simulink model "TosAeb"  (R2021a)
settings: Solver=FixedStepDiscrete  StartTime=0.0  StopTime=inf  FixedStep=0.01  RelTol=1e-3
undefined symbols (not defined in the file or in its folder): ACCModSts, AccSts1, AEB_ObjectMovingOrientation, AEB_ObjectMovingState, CN_BTCActive, CN_CurrentGearR, CN_CurrentGearR_DHT, CN_PTCActive, CN_VDCActive, CN_VSC_EspOff, CN_VSC_FCW_AEB_Error, CN_VSC_FCW_AEB_NoDispay, CN_VSC_FCW_AEB_PerfmDgdtn, CN_VSC_FCW_AEB_TempUnAvlbl, CN_VSC_VehStandstill, CNaccelAvoidMax, CNaccelAvoidMin, CNbrakePedalPress, CNcomfortStrLeftAccelMax, CNcomfortStrLeftAccelMin, CNcomfortStrRightAccelMax, CNcomfortStrRightAccelMin, CNDrvDoorOpen, CNDrvModeEco, CNDrvModeNorm, CNDrvModeOther, CNDrvModeSport, CNDrvSBRreminder, CNNull, CNobjInvalidID, CNPassDoorOpen, CNPassSBRreminder, CNPEBSAccelNegInf, CNPEBSAccelNull, CNPEBSTimeInf, CNPEBSTimeNull, CNSTOPMAX, CNSTOPNULL, CNsubjWidth, CNSwtOFF, CNSwtON, CNTTCMAX, CNTTCNULL, CNVehConfigAEBCycNone, CNVehConfigAEBNone, CNVehConfigAEBPedNone, CNVehConfigFCWNone, CNVehConfigJANone, CollisionSeverity, FALSE, FCWSnvty, JACycObjCalcInfoDefaultParams, JAPedObjCalcInfoDefaultParams, JAVehObjCalcInfoDefaultParams, latCycObjCalcInfoDefaultParams, latPedObjCalcInfoDefaultParams, longObjCalcInfoDefaultParams, ModSts, movingStatus, ObjectType ... (+1112)
libraries: simulink x3031, AEBSFunctionLib x50, Ta_Library x33, AEBSStateMachineLib x23, Mylibrary x12, simulink_extras x7, ObjSelect_lib x3, dspstat3 x2
(part 81 of 102)

== system TosAeb/AEB_Objsel/TA_Processor/AEB_ObjSelect/TargetInfoCheck/Data_precess/TA_EffectCalculate/Obj_FilterFlgCalculate/Lat_ObjFilter/Lat_Objelse/Lat_ObjPre/Subsystem/LatDis_Cal/Width_Calculate ==
  Width : Inport
  Add : Sum
  Constant : Constant  Value=0.5
  Constant1 : Constant  Value=P_TA_PrmForVehWidth
  Product : Product
  Product1 : Product
  Width_Large : Outport
  Width_half : Outport  Port=2
  wires:
  Constant/1 -> Product/2, Product1/1
  Product1/1 -> Width_half/1, Add/2
  Product/1 -> Add/1
  Constant1/1 -> Product1/2
  Width/1 -> Product/1
  Add/1 -> Width_Large/1

== system TosAeb/AEB_Objsel/TA_Processor/AEB_ObjSelect/TargetInfoCheck/Data_precess/TA_EffectCalculate/Obj_FilterFlgCalculate/Lat_ObjFilter/Lat_Objelse/Lat_ObjPre/Subsystem/LatDis_Cal/others ==
  Action Port : ActionPort  ActionPortLabel=else
  Constant : Constant  Value=0  OutDataTypeStr=boolean
  Constant1 : Constant  Value=100  OutDataTypeStr=single
  Valid_Flg : Outport
  TTI : Outport  Port=2
  wires:
  Constant1/1 -> TTI/1
  Constant/1 -> Valid_Flg/1

== system TosAeb/AEB_Objsel/TA_Processor/AEB_ObjSelect/TargetInfoCheck/Data_precess/TA_EffectCalculate/Obj_FilterFlgCalculate/Lat_ObjFilter/Lat_Objelse/Lat_ObjPre/Subsystem/LatDis_Cal1/L2R ==
  dy : Inport
  Width_Large : Inport  Port=2
  Width_half : Inport  Port=3
  dx : Inport  Port=4
  ax : Inport  Port=5
  ay : Inport  Port=6
  vx : Inport  Port=7
  vy : Inport  Port=8
  Action Port : ActionPort
  Constant : Constant  Value=P_TA_PrmForVehLongth
  L2R_Calculate : MATLAB Function  TreatAsAtomicUnit=on
  Valid_Flg : Outport
  TTI : Outport  Port=2
  wires:
  Constant/1 -> L2R_Calculate/9(P_TA_PrmForVehLongth)
  L2R_Calculate/2(TTI) -> TTI/1
  L2R_Calculate/1(Valid_Flg) -> Valid_Flg/1
  Width_half/1 -> L2R_Calculate/3(Width_half)
  dx/1 -> L2R_Calculate/4(dx)
  Width_Large/1 -> L2R_Calculate/2(Width_Large)
  ax/1 -> L2R_Calculate/5(ax)
  dy/1 -> L2R_Calculate/1(dy)
  vy/1 -> L2R_Calculate/8(vy)
  ay/1 -> L2R_Calculate/6(ay)
  vx/1 -> L2R_Calculate/7(vx)

== system TosAeb/AEB_Objsel/TA_Processor/AEB_ObjSelect/TargetInfoCheck/Data_precess/TA_EffectCalculate/Obj_FilterFlgCalculate/Lat_ObjFilter/Lat_Objelse/Lat_ObjPre/Subsystem/LatDis_Cal1/Obj_PosnJudge ==
  Oreintation : Inport
  vy : Inport  Port=2
  Compare To Constant2 : simulink/Logic and Bit Operations/Compare To Constant  relop===  const=3  OutDataTypeStr=boolean
  Compare To Constant3 : simulink/Logic and Bit Operations/Compare To Constant  relop=>  const=P_TA_PrmForLatObjLatSpdThred  OutDataTypeStr=boolean
  Compare To Constant4 : simulink/Logic and Bit Operations/Compare To Constant  relop===  const=2  OutDataTypeStr=boolean
  Compare To Constant5 : simulink/Logic and Bit Operations/Compare To Constant  relop=>  const=P_TA_PrmForLatObjLatSpdThred  OutDataTypeStr=boolean
  Data Type Conversion : DataTypeConversion
  Data Type Conversion1 : DataTypeConversion
  Gain1 : Gain  Gain=-1  OutDataTypeStr=single
  Ground_1 : Ground
  Logical Operator : Logic  AllPortsSameDT=off  OutDataTypeStr=boolean
  Logical Operator1 : Logic  AllPortsSameDT=off  OutDataTypeStr=boolean
  Terminator_1 : Terminator
  L2R : Outport
  R2L : Outport  Port=2
  wires:
  Gain1/1 -> Terminator_1/1
  Ground_1/1 -> Gain1/1
  Data Type Conversion1/1 -> R2L/1
  Data Type Conversion/1 -> L2R/1
  Logical Operator/1 -> Data Type Conversion/1
  Logical Operator1/1 -> Data Type Conversion1/1
  Compare To Constant3/1 -> Logical Operator/2
  vy/1 -> Compare To Constant3/1, Compare To Constant5/1
  Compare To Constant4/1 -> Logical Operator1/1
  Compare To Constant5/1 -> Logical Operator1/2
  Compare To Constant2/1 -> Logical Operator/1
  Oreintation/1 -> Compare To Constant2/1, Compare To Constant4/1

== system TosAeb/AEB_Objsel/TA_Processor/AEB_ObjSelect/TargetInfoCheck/Data_precess/TA_EffectCalculate/Obj_FilterFlgCalculate/Lat_ObjFilter/Lat_Objelse/Lat_ObjPre/Subsystem/LatDis_Cal1/R2L ==
  dy : Inport
  Width_Large : Inport  Port=2
  Width_half : Inport  Port=3
  dx : Inport  Port=4
  ax : Inport  Port=5
  ay : Inport  Port=6
  vx : Inport  Port=7
  vy : Inport  Port=8
  Action Port : ActionPort
  Constant : Constant  Value=P_TA_PrmForVehLongth
  R2L_Calculate : MATLAB Function  TreatAsAtomicUnit=on
  Valid_Flg : Outport
  TTI : Outport  Port=2
  wires:
  Constant/1 -> R2L_Calculate/9(P_TA_PrmForVehLongth)
  R2L_Calculate/2(TTI) -> TTI/1
  R2L_Calculate/1(Valid_Flg) -> Valid_Flg/1
  vx/1 -> R2L_Calculate/7(vx)
  ay/1 -> R2L_Calculate/6(ay)
  vy/1 -> R2L_Calculate/8(vy)
  dy/1 -> R2L_Calculate/1(dy)
  ax/1 -> R2L_Calculate/5(ax)
  Width_Large/1 -> R2L_Calculate/2(Width_Large)
  dx/1 -> R2L_Calculate/4(dx)
  Width_half/1 -> R2L_Calculate/3(Width_half)

== system TosAeb/AEB_Objsel/TA_Processor/AEB_ObjSelect/TargetInfoCheck/Data_precess/TA_EffectCalculate/Obj_FilterFlgCalculate/Lat_ObjFilter/Lat_Objelse/Lat_ObjPre/Subsystem/LatDis_Cal1/Width_Calculate ==
  Width : Inport
  Add : Sum
  Constant : Constant  Value=0.5
  Constant1 : Constant  Value=P_TA_PrmForVehWidth
  Product : Product
  Product1 : Product
  Width_Large : Outport
  Width_half : Outport  Port=2
  wires:
  Add/1 -> Width_Large/1
  Width/1 -> Product/1
  Constant1/1 -> Product1/2
  Product/1 -> Add/1
  Product1/1 -> Add/2, Width_half/1
  Constant/1 -> Product1/1, Product/2

== system TosAeb/AEB_Objsel/TA_Processor/AEB_ObjSelect/TargetInfoCheck/Data_precess/TA_EffectCalculate/Obj_FilterFlgCalculate/Lat_ObjFilter/Lat_Objelse/Lat_ObjPre/Subsystem/LatDis_Cal1/others ==
  Action Port : ActionPort
  Constant : Constant  Value=0  OutDataTypeStr=boolean
  Constant1 : Constant  Value=100  OutDataTypeStr=single
  Valid_Flg : Outport
  TTI : Outport  Port=2
  wires:
  Constant/1 -> Valid_Flg/1
  Constant1/1 -> TTI/1

== system TosAeb/AEB_Objsel/TA_Processor/AEB_ObjSelect/TargetInfoCheck/Data_precess/TA_EffectCalculate/Obj_FilterFlgCalculate/Long_ObjFilter/OverlapCalculate/BoudingBoxCalculate_Else/Overlap_FlgCalculate/AbsPosn_InsectionFlg/ObjBoundingBox ==
  ObjLength : Inport  OutDataTypeStr=single
  ObjWidth : Inport  Port=2  OutDataTypeStr=single
  dxPreRefPointObj : Inport  Port=3  OutDataTypeStr=single
  dyPreRefPointObj : Inport  Port=4  OutDataTypeStr=single
  sincosCoef : Inport  Port=5
  Bus Creator : BusCreator  Inputs=5
  Mux : Mux
  Mux1 : Mux
  ObjLLCoordinate : Subsystem
  ObjLUCoordinate : Subsystem
  ObjRLCoordinate : Subsystem
  ObjRUCoordinate : Subsystem
  objDxPre : Outport
  objDyPre : Outport  Port=2
  wires:
  ObjLength/1 -> Bus Creator/1  "ObjLength"
  ObjWidth/1 -> Bus Creator/2  "ObjWidth"
  dxPreRefPointObj/1 -> Bus Creator/3  "dxPreRefPointObj"
  dyPreRefPointObj/1 -> Bus Creator/4  "dyPreRefPointObj"
  sincosCoef/1 -> Bus Creator/5  "sincosCoef"
  Bus Creator/1 -> ObjRLCoordinate/1(inputSignals), ObjRUCoordinate/1(inputSignals), ObjLLCoordinate/1(inputSignals), ObjLUCoordinate/1(inputSignals)
  ObjLUCoordinate/1(dxPreLUObj) -> [dxPreLUObj]  "dxPreLUObj"
  ObjLUCoordinate/2(dyPreLUobj) -> [dyPreLUObj]  "dyPreLUObj"
  ObjLLCoordinate/1(dxPre_LLObj) -> [dxPreLLObj]  "dxPreLLObj"
  ObjLLCoordinate/2(dyPreLLObj) -> [dyPreLLObj]  "dyPreLLObj"
  ObjRUCoordinate/1(dxPreRUObj) -> [dxPreRUObj]  "dxPreRUObj"
  ObjRUCoordinate/2(dyPreRUObj) -> [dyPreRUObj]  "dyPreRUObj"
  ObjRLCoordinate/1(dxPreRLObj) -> [dxPreRLObj]  "dxPreRLObj"
  ObjRLCoordinate/2(dyPreRLObj) -> [dyPreRLObj]  "dyPreRLObj"
  Mux/1 -> objDxPre/1
  Mux1/1 -> objDyPre/1
  [dxPreRUObj] -> Mux/1  "dxPreRUObj"
  [dxPreLUObj] -> Mux/2
  [dxPreLLObj] -> Mux/3
  [dxPreRLObj] -> Mux/4
  [dyPreRUObj] -> Mux1/1
  [dyPreLUObj] -> Mux1/2
  [dyPreLLObj] -> Mux1/3
  [dyPreRLObj] -> Mux1/4

== system TosAeb/AEB_Objsel/TA_Processor/AEB_ObjSelect/TargetInfoCheck/Data_precess/TA_EffectCalculate/Obj_FilterFlgCalculate/Long_ObjFilter/OverlapCalculate/BoudingBoxCalculate_Else/Overlap_FlgCalculate/AbsPosn_InsectionFlg/Overlap_Judgment ==
  subjDxPre : Inport
  subjDyPre : Inport  Port=2
  objDxPre : Inport  Port=3
  objDyPre : Inport  Port=4
  MinMax{,2,4,6} (4 alike) : MinMax
  MinMax{1,3,5,7} (4 alike) : MinMax  Function=max
  Overlap_FlgCalculate : MATLAB Function  TreatAsAtomicUnit=on
  Abs_OverlapFlg : Outport
  wires:
  subjDxPre/1 -> MinMax1/1, MinMax/1
  subjDyPre/1 -> MinMax2/1, MinMax3/1
  objDxPre/1 -> MinMax4/1, MinMax5/1
  objDyPre/1 -> MinMax6/1, MinMax7/1
  MinMax3/1 -> Overlap_FlgCalculate/3(Obj_y_Max)
  MinMax2/1 -> Overlap_FlgCalculate/4(Obj_y_Min)
  MinMax1/1 -> Overlap_FlgCalculate/1(Obj_x_Max)
  MinMax/1 -> Overlap_FlgCalculate/2(Obj_x_Min)
  MinMax5/1 -> Overlap_FlgCalculate/5(Subj_x_Max)
  MinMax4/1 -> Overlap_FlgCalculate/6(Subj_x_Min)
  MinMax7/1 -> Overlap_FlgCalculate/7(Subj_y_Max)
  MinMax6/1 -> Overlap_FlgCalculate/8(Subj_y_Min)
  Overlap_FlgCalculate/1(Overlap_Flg) -> Abs_OverlapFlg/1

== system TosAeb/AEB_Objsel/TA_Processor/AEB_ObjSelect/TargetInfoCheck/Data_precess/TA_EffectCalculate/Obj_FilterFlgCalculate/Long_ObjFilter/OverlapCalculate/BoudingBoxCalculate_Else/Overlap_FlgCalculate/AbsPosn_InsectionFlg/SubjBoundingBox ==
  dxPreRefPoint : Inport  OutDataTypeStr=single
  dyPreRefPoint : Inport  Port=2  OutDataTypeStr=single
  sincosCoef : Inport  Port=3
  Bus Creator : BusCreator  Inputs=8
  Constant : Constant  Value=P_TA_PrmForVehRearAxleDisttoFrnt  OutDataTypeStr=single
  Constant1 : Constant  Value=P_TA_PrmForVehRearAxleDisttoRear  OutDataTypeStr=single
  Constant2 : Constant  Value=P_TA_PrmForVehWidth  OutDataTypeStr=single
  Constant3 : Constant  Value=P_TA_PrmForVehRearAxleDisttoFrnt  OutDataTypeStr=single
  Mux : Mux
  Mux1 : Mux
  SubjLLCoordinate : Subsystem
  SubjLUCoordinate : Subsystem
  SubjRLCoordinate : Subsystem
  SubjRUCoordinate : Subsystem
  Subsystem : Subsystem
  subjDxPre : Outport
  subjDyPre : Outport  Port=2
  wires:
  Constant2/1 -> Bus Creator/6  "subjWidth"
  Constant1/1 -> Bus Creator/5  "dxRearBumperToRearAxis"
  Constant/1 -> Bus Creator/4  "dxFrontBumperToRearAxis"
  sincosCoef/1 -> [Coeficence], Bus Creator/3  "sincosCoef"
  dyPreRefPoint/1 -> Bus Creator/2  "dyPreRefPoint"
  dxPreRefPoint/1 -> Bus Creator/1  "dxPreRefPoint"
  Bus Creator/1 -> SubjLUCoordinate/1(inputSignals), SubjRLCoordinate/1(inputSignals), SubjRUCoordinate/1(inputSignals), SubjLLCoordinate/1(inputSignals)
  SubjLUCoordinate/1(dxPreLU) -> [dxPreLU]  "dxPreLU"
  SubjLUCoordinate/2(dyPreLU) -> [dyPreLU]  "dyPreLU"
  SubjLLCoordinate/1(dxPreLL) -> [dxPreLL]  "dxPreLL"
  SubjLLCoordinate/2(dyPreLL) -> [dyPreLL]  "dyPreLL"
  SubjRUCoordinate/1(dxPreRU) -> [dxPreRU]  "dxPreRU"
  SubjRUCoordinate/2(dyPreRU) -> [dyPreRU]  "dyPreRU"
  SubjRLCoordinate/1(dxPreRL) -> [dxPreRL]  "dxPreRL"
  SubjRLCoordinate/2(dyPreRL) -> [dyPreRL]  "dyPreRL"
  Mux/1 -> subjDxPre/1
  Mux1/1 -> subjDyPre/1
  [dxPreRU] -> Mux/1
  [dxPreLU] -> Mux/2
  [dxPreLL] -> Mux/3
  [dxPreRL] -> Mux/4
  [dyPreRU] -> Mux1/1
  [dyPreLU] -> Mux1/2
  [dyPreLL] -> Mux1/3
  [dyPreRL] -> Mux1/4
  Constant3/1 -> Subsystem/1(dxFrontBumperToRearAxis)
  [Coeficence] -> Subsystem/2(sincosCoef)
  Subsystem/1(Lat_Offset) -> Bus Creator/7
  Subsystem/2(Long_Offset) -> Bus Creator/8

== system TosAeb/AEB_Objsel/TA_Processor/AEB_ObjSelect/TargetInfoCheck/Data_precess/TA_EffectCalculate/Obj_FilterFlgCalculate/Long_ObjFilter/OverlapCalculate/BoudingBoxCalculate_Else/Overlap_FlgCalculate/AbsPosn_InsectionFlg/sincosCoefCalc ==
  angle : Inport  OutDataTypeStr=single
  Bus Creator : BusCreator  Inputs=2
  Sincos : Trigonometry  Operator=sincos
  sincosCoef : Outport
  wires:
  Sincos/1 -> Bus Creator/1  "sinCoef"
  angle/1 -> Sincos/1
  Sincos/2 -> Bus Creator/2  "cosCoef"
  Bus Creator/1 -> sincosCoef/1  "sincosCoef"

== system TosAeb/AEB_Objsel/TA_Processor/AEB_ObjSelect/TargetInfoCheck/Data_precess/TA_EffectCalculate/Obj_FilterFlgCalculate/Long_ObjFilter/OverlapCalculate/BoudingBoxCalculate_Else/Overlap_FlgCalculate/AbsPosn_InsectionFlg/sincosCoefCalc1 ==
  angle : Inport  OutDataTypeStr=single
  Bus Creator : BusCreator  Inputs=2
  Sincos : Trigonometry  Operator=sincos
  sincosCoef : Outport
  wires:
  Bus Creator/1 -> sincosCoef/1  "sincosCoef"
  Sincos/2 -> Bus Creator/2  "cosCoef"
  angle/1 -> Sincos/1
  Sincos/1 -> Bus Creator/1  "sinCoef"

== system TosAeb/AEB_Objsel/TA_Processor/AEB_ObjSelect/TargetInfoCheck/Data_precess/TA_EffectCalculate/Obj_FilterFlgCalculate/Long_ObjFilter/OverlapCalculate/BoudingBoxCalculate_Else/PosnatTTI/LongitudinalMotionData/Subsystem1 ==
  In1 : Inport
  In2 : Inport  Port=2
  In3 : Inport  Port=3
  In4 : Inport  Port=4
  TA_ObjInfo : Inport  Port=5
  In6 : Inport  Port=6
  u : Inport  Port=7
  VehSelfData : Inport  Port=8
  Add : Sum
  Add1 : Sum
  Atan2 : Trigonometry  Operator=atan2
  Bus Selector2 : BusSelector  OutputSignals=TA_ObjAccel,TA_Objvx,TA_Objvy,TA_Objdx,TA_ObjLength,TA_Objdy,TA_ObjState
  Bus Selector3 : BusSelector  OutputSignals=VehSelf.Veh_DriverAcc,VehSelf.YawRate,VehSelf.Veh_LongSpd_mps
  Compare To Constant : simulink/Logic and Bit Operations/Compare To Constant  relop===  const=0  OutDataTypeStr=boolean
  Compare To Constant1 : simulink/Logic and Bit Operations/Compare To Constant  relop===  const=1  OutDataTypeStr=boolean
  Constant : Constant  Value=pi/2  OutDataTypeStr=single
  Constant16 : Constant  Value=P_TA_PrmForVehRearAxleDisttoFrnt
  Logical Operator : Logic  Operator=OR  AllPortsSameDT=off  OutDataTypeStr=boolean
  MapToAngularInterval : Ta_Library/MapToAngularInterval  SystemSampleTime=-1  Latency=0  AutoFrameSizeCalculation=off
  Obj_InfoCal : Subsystem
  Product : Product
  Subj_AyCal : Subsystem
  Terminator{,1..4} (5 alike) : Terminator
  <TA_ObjAccel> : Outport
  Ref_Vx : Outport  Port=2
  <TA_Objvx> : Outport  Port=3
  Ref_Vy : Outport  Port=4
  <TA_Objvy> : Outport  Port=5
  Obj_dx : Outport  Port=6
  <TA_Objdx> : Outport  Port=7
  Obj_dy : Outport  Port=8
  <TA_Objdy> : Outport  Port=9
  <TA_ObjState> : Outport  Port=10
  Obj_Vy : Outport  Port=11
  Obj_Ax : Outport  Port=12
  Obj_Ay : Outport  Port=13
  Ref_ax : Outport  Port=14
  Ref_ay : Outport  Port=15
  Obj_Vx : Outport  Port=16
  wires:
  In1/1 -> [Obj_Acc]
  In4/1 -> Obj_dx/1
  Bus Selector2/4 -> <TA_Objdx>/1  "<TA_Objdx>"
  Constant/1 -> MapToAngularInterval/2
  Obj_InfoCal/1(Obj_AbsAcclx) -> Obj_Ax/1
  MapToAngularInterval/1 -> Obj_InfoCal/5(MappedAngle)
  Bus Selector3/1 -> [Acc_x], Terminator/1  "<Veh_DriverAcc>"
  [Obj_Vx] -> Add/1
  VehSelfData/1 -> Bus Selector3/1
  In2/1 -> [Obj_Vx], Ref_Vx/1
  TA_ObjInfo/1 -> Bus Selector2/1
  [Obj_Acc] -> Obj_InfoCal/2(TA_ObjAccel)
  [Acc_x] -> Subj_AyCal/3(ACC_x)
  In6/1 -> Obj_dy/1
  [stop] -> Obj_InfoCal/1(stop)
  Logical Operator/1 -> [stop]
  Compare To Constant1/1 -> Logical Operator/2
  Add1/1 -> Atan2/1, Obj_Vy/1
  Obj_InfoCal/4(Ref_ay) -> Ref_ay/1
  Bus Selector2/1 -> <TA_ObjAccel>/1  "<TA_ObjAccel>"
  In3/1 -> [Obj_vy], Ref_Vy/1
  Bus Selector2/2 -> <TA_Objvx>/1  "<TA_Objvx>"
  Bus Selector2/5 -> Terminator4/1  "<TA_ObjLength>"
  Compare To Constant/1 -> Logical Operator/1
  Constant16/1 -> Product/2
  Subj_AyCal/1(Subj_Ay) -> [Acc_y], Terminator3/1
  Bus Selector2/3 -> <TA_Objvy>/1  "<TA_Objvy>"
  Obj_InfoCal/3(Ref_ax) -> Ref_ax/1
  Bus Selector3/3 -> Subj_AyCal/1(Subj_Vx), Terminator1/1, Add/2  "<Veh_LongSpd_mps>"
  [Subj_Vy] -> Subj_AyCal/2(Subj_Vy)
  Add/1 -> Atan2/2, Obj_Vx/1
  Bus Selector3/2 -> Product/1  "<YawRate>"
  Bus Selector2/6 -> <TA_Objdy>/1  "<TA_Objdy>"
  [Acc_x] -> Obj_InfoCal/3(Subj_Ax)
  Obj_InfoCal/2(Obj_AbsAccly) -> Obj_Ay/1
  [Subj_Vy] -> Terminator2/1
  Bus Selector2/7 -> <TA_ObjState>/1  "<TA_ObjState>"
  [Acc_y] -> Obj_InfoCal/4(Subj_Ay)
  u/1 -> Compare To Constant/1, Compare To Constant1/1
  [Obj_vy] -> Add1/1
  Atan2/1 -> MapToAngularInterval/1
  Product/1 -> Add1/2, [Subj_Vy]

== system TosAeb/AEB_Objsel/TA_Processor/AEB_ObjSelect/TargetInfoCheck/Data_precess/TA_EffectCalculate/Obj_FilterFlgCalculate/Long_ObjFilter/OverlapCalculate/BoudingBoxCalculate_Else/PosnatTTI/PredictHostPositionAtTTR/CalculateHeading ==
  note: If the current point is in segment i, and the speed is close to zero,
      | then the speed at the start point of segment i, i-1,...,1 are checked for non-zero speed.
      | The first non-zero speed found is used as basis for calculating the heading angle.
      | If all checked points have zero speed, then the heading is set to zero.
  PathEstimn : Inport
  SegmentIndex : Inport  Port=2
  dx : Inport  Port=3
  dy : Inport  Port=4
  CheckSpeed : Subsystem
  ExtractVelocitiesAtStartOfSegments : Subsystem  TreatAsAtomicUnit=on
  FindValidIndex : MATLAB Function  TreatAsAtomicUnit=on
  FindValidVelocityVector : MATLAB Function  TreatAsAtomicUnit=on
  atan2 : Trigonometry  Operator=atan2
  PredHostHeading : Outport
  wires:
  ExtractVelocitiesAtStartOfSegments/3(SegmentVelocitiesY) -> FindValidVelocityVector/6(SegmentVelocitiesY)
  ExtractVelocitiesAtStartOfSegments/2(SegmentVelocitiesX) -> FindValidVelocityVector/5(SegmentVelocitiesX)
  ExtractVelocitiesAtStartOfSegments/1(SpeedValid) -> FindValidIndex/2(SpeedValid)
  FindValidVelocityVector/1(ValidVelocityY) -> atan2/1
  FindValidVelocityVector/2(ValidVelocityX) -> atan2/2
  CheckSpeed/1(SpeedValid) -> FindValidVelocityVector/1(CurrentVelocityValid)
  FindValidIndex/1(ValidSegmentIndex) -> FindValidVelocityVector/2(ValidSegmentIndex)
  [dx] -> FindValidVelocityVector/3(CurrentVelocityX)
  [dy] -> FindValidVelocityVector/4(CurrentVelocityY)
  SegmentIndex/1 -> FindValidIndex/1(SegmentIndex)
  atan2/1 -> PredHostHeading/1  "PredHostHeading"
  PathEstimn/1 -> ExtractVelocitiesAtStartOfSegments/1(PathEstimn)
  dx/1 -> CheckSpeed/1(x), [dx]
  dy/1 -> CheckSpeed/2(y), [dy]

== system TosAeb/AEB_Objsel/TA_Processor/AEB_ObjSelect/TargetInfoCheck/Data_precess/TA_EffectCalculate/Obj_FilterFlgCalculate/Long_ObjFilter/OverlapCalculate/BoudingBoxCalculate_Else/PosnatTTI/PredictHostPositionAtTTR/HeadingCalculate ==
  PredictionTime : Inport
  VehSelfData : Inport  Port=2
  Bus Selector : BusSelector  OutputSignals=VehSelf.YawRate,Curvature
  Product : Product
  Curvatrue : Outport
  Heanding : Outport  Port=2
  wires:
  Bus Selector/1 -> Product/2  "<YawRate>"
  Product/1 -> Heanding/1
  VehSelfData/1 -> Bus Selector/1
  PredictionTime/1 -> Product/1
  Bus Selector/2 -> Curvatrue/1  "<Curvature>"

== system TosAeb/AEB_Objsel/TA_Processor/AEB_ObjSelect/TargetInfoCheck/Data_precess/TA_EffectCalculate/Obj_FilterFlgCalculate/Long_ObjFilter/OverlapCalculate/BoudingBoxCalculate_Else/PosnatTTI/PredictHostPositionAtTTR/SegmentComponents ==
  note: 判断预测时间的位置，与第一段时间做差，然后除以第一段与第四段的差值，输出数
      | 值为0到1的数
  note: 驾驶员预测前方道路分为4段，输出为每段的4个点的横纵坐标值，然后做拟合处理
  Segment : Inport
  PredictionTime : Inport  Port=2
  Curvatrue : Inport  Port=3
  Abs : Abs
  Add1 : Sum  Inputs=+-
  Bus Selector : BusSelector  OutputSignals=CtrlAryLgt,CtrlAryLat,TiAry
  Compare To Constant : simulink/Logic and Bit Operations/Compare To Constant  relop=<=  const=0.001  OutDataTypeStr=boolean
  Logical Operator : Logic  Operator=NOT  AllPortsSameDT=off  OutDataTypeStr=boolean
  SafeDivide : Subsystem  TreatAsAtomicUnit=on  mask(Safe divide){DataType=single, DataTypeMax=0, DataTypeMin=0}
      mask prompts: DataType "Data type:"
      mask help:
      | This block offers division protected from division by zero.
      |
      | When division by zero is detected, a very large number will be returned with the same sign as the numerator.
      |
      | In the case zero divided by zero, the output has been defined as zero.
      |
      | Both floating-point numbers and integers are supported.
      mask init: same as TosAeb/AEB_Objsel/TA_Processor/PreProcessor/VehiclePreProcessor/CalculateCurvature/SafeDivide
      settings: RTWSystemCode=Inline
      mask Help:
      | This block offers division protected from division by zero.
      | 
      | When division by zero is detected, a very large number will be returned with the same sign as the numerator.
      | 
      | In the case zero divided by zero, the output has been defined as zero.
      | 
      | Both floating-point numbers and integers are supported.
      mask Display:
      | % Author: Jakob Andersson (jande573)
      | 
      | % Display the allowed data type
      | disp(DataType);
      | 
      | % Display the port symbols
      | port_label('input',1,'\bullet','texmode','on');
      | port_label('input',2,'\div','texmode','on');
  Saturation : Saturate  UpperLimit=1  LowerLimit=0  OutDataTypeStr=single
      settings: AttributesFormatString=LowerLimit=%<LowerLimit>\nUpperLimit=%<UpperLimit>
  Saturation1 : Saturate  UpperLimit=inf  LowerLimit=single(0.001)  OutDataTypeStr=single
      settings: AttributesFormatString=LowerLimit=%<LowerLimit>\nUpperLimit=%<UpperLimit>
  Selector : Selector  InputPortWidth=4  IndexOptions=Index vector (dialog)  Indices=1  OutputSizes=1
  Selector1 : Selector  InputPortWidth=4  IndexOptions=Index vector (dialog)  Indices=2  OutputSizes=1
  Selector2 : Selector  InputPortWidth=4  IndexOptions=Index vector (dialog)  Indices=3  OutputSizes=1
  Selector3 : Selector  InputPortWidth=4  IndexOptions=Index vector (dialog)  Indices=4  OutputSizes=1
  Selector4 : Selector  InputPortWidth=4  IndexOptions=Index vector (dialog)  Indices=1  OutputSizes=1
  Selector5 : Selector  InputPortWidth=4  IndexOptions=Index vector (dialog)  Indices=2  OutputSizes=1
  Selector6 : Selector  InputPortWidth=4  IndexOptions=Index vector (dialog)  Indices=3  OutputSizes=1
  Selector7 : Selector  InputPortWidth=4  IndexOptions=Index vector (dialog)  Indices=4  OutputSizes=1
  Selector8 : Selector  InputPortWidth=2  IndexOptions=Index vector (dialog)  Indices=1  OutputSizes=1
  Selector9 : Selector  InputPortWidth=2  IndexOptions=Index vector (dialog)  Indices=2  OutputSizes=1
  Terminator : Terminator
  time duration : Sum  Inputs=-+
  P0x : Outport
  P1x : Outport  Port=2
  P2x : Outport  Port=3
  P3x : Outport  Port=4
  P0y : Outport  Port=5
  P1y : Outport  Port=6
  P2y : Outport  Port=7
  P3y : Outport  Port=8
  tau : Outport  Port=9
  wires:
  Saturation/1 -> tau/1  "tau"
  SafeDivide/1(Quotient) -> Saturation/1  "RelativeTime"
  time duration/1 -> Saturation1/1
  Add1/1 -> SafeDivide/1(Numerator)
  PredictionTime/1 -> Add1/1
  Selector8/1 -> Add1/2, time duration/1  "TimeStart"
  Selector9/1 -> time duration/2  "TimeEnd"
  Bus Selector/3 -> Selector9/1, Selector8/1  "<TiAry>"
  Bus Selector/2 -> Selector5/1, Selector6/1, Selector7/1, Selector4/1  "<CtrlAryLat>"
  Selector7/1 -> P3y/1  "P3y"
  Selector6/1 -> P2y/1  "P2y"
  Selector5/1 -> P1y/1  "P1y"
  Selector4/1 -> P0y/1  "P0y"
  Selector3/1 -> P3x/1  "P3x"
  Selector2/1 -> P2x/1  "P2x"
  Selector1/1 -> P1x/1  "P1x"
  Selector/1 -> P0x/1  "P0x"
  Bus Selector/1 -> Selector2/1, Selector1/1, Selector/1, Selector3/1  "<CtrlAryLgt>"
  Segment/1 -> Bus Selector/1
  Saturation1/1 -> SafeDivide/2(Denominator)
  Curvatrue/1 -> Abs/1
  Abs/1 -> Compare To Constant/1
  Compare To Constant/1 -> Logical Operator/1
  Logical Operator/1 -> Terminator/1

== system TosAeb/AEB_Objsel/TA_Processor/AEB_ObjSelect/TargetInfoCheck/Data_precess/TA_EffectCalculate/Obj_FilterFlgCalculate/Long_ObjFilter/OverlapCalculate/BoudingBoxCalculate_Else/PosnatTTI/PredictHostPositionAtTTR/SelectSegment ==
  note: The purpose is to choose that segment such that:
      | Path.T1 < Time <= Path.T2
      | However if Time is large, i.e. Time > Path.T2
      | for all Path.Segments then choose last Path
  note: Maximum one element can be true
      | in the TimeWithinSegment vector.
  PahEstimn : Inport  OutDataTypeStr=Bus: PahEstimn
  Time : Inport  Port=2
  Constant : Constant  OutDataTypeStr=uint8
  ForEachSegmentInPahEstimn : Subsystem  TreatAsAtomicUnit=on
  Indices : Constant  Value=uint8([1:4])
  Logop_1 : Logic  Operator=OR  Inputs=1  AllPortsSameDT=off  OutDataTypeStr=boolean
  Product : Product  RndMeth=Zero
  Selector : Selector  IndexOptions=Index vector (port)  Indices=[1 3]  OutputSizes=1
  Selector1 : Selector  IndexOptions=Index vector (port)  Indices=[1 3]  OutputSizes=1
  Sum of Elements : Sum  Inputs=+
  Switch : Switch  Criteria=u2 ~= 0
  Width : Width  DataType=uint8
  Segment : Outport
  SegmentIndex : Outport  Port=2
  Segment1 : Outport  Port=3
  wires:
  PahEstimn/1 -> ForEachSegmentInPahEstimn/1(PahEstimn), Selector1/1, Selector/1, [PahEstimn]
  Time/1 -> ForEachSegmentInPahEstimn/2(Time)
  Switch/1 -> SegmentIndex/1, Selector/2  "SegmentIndex"
  Selector/1 -> Segment/1  "Segment"
  ForEachSegmentInPahEstimn/1(TimeWithinSegment) -> Logop_1/1, Product/2
  Logop_1/1 -> Switch/2  "OneSegmentFound"
  Width/1 -> Switch/3  "LastSegment"
  Sum of Elements/1 -> Switch/1  "IndexOfSegment"
  [PahEstimn] -> Width/1
  Product/1 -> Sum of Elements/1  "IndexVector"
  Indices/1 -> Product/1
  Constant/1 -> Selector1/2
  Selector1/1 -> Segment1/1  "Segment"

== system TosAeb/AEB_Objsel/TA_Processor/AEB_ObjSelect/TargetInfoCheck/Data_precess/TA_EffectCalculate/Obj_FilterFlgCalculate/Long_ObjFilter/OverlapCalculate/BoudingBoxCalculate_Else/PosnatTTI/PredictObjectPositionAtTTR/LP ==
  Raw : Inport
  Constant2 : Constant  Value=0.02  OutDataTypeStr=single
  FilterTime1 : Constant  Value=0.12  OutDataTypeStr=single
  Product2 : Product  Inputs=*/  RndMeth=Zero
  Product6 : Product  RndMeth=Zero
  Sum6 : Sum  Inputs=+-
  Sum7 : Sum
  Unit Delay : UnitDelay  SampleTime=-1  HasFrameUpgradeWarning=on
  ADS_EngTorqReq : Outport  VectorParamsAs1DForOutWhenUnconnected=off
  wires:
  Raw/1 -> Sum6/1
  Product6/1 -> Sum7/1
  Product2/1 -> Product6/1
  Sum6/1 -> Product6/2
  FilterTime1/1 -> Product2/2
  Constant2/1 -> Product2/1
  Unit Delay/1 -> Sum7/2, Sum6/2
  Sum7/1 -> Unit Delay/1, ADS_EngTorqReq/1

== system TosAeb/AEB_Objsel/TA_Processor/AEB_ObjSelect/TargetInfoCheck/Data_precess/TA_EffectCalculate/Obj_FilterFlgCalculate/Long_ObjFilter/TTR_Calculate/CalculateTTRAndTTM_else/LongitudinalTTRComputer/CalculateTTI/HostAndObjectMoving ==
  note: 100
  EdgeDistanceNormalSetup : Inport
  MotionDataNormalSetup : Inport  Port=2
  EdgeDistanceAlternativeSetup : Inport  Port=3
  MotionDataAlternativeSetup : Inport  Port=4
  AEB_State : Inport  Port=5
  Bus Selector : BusSelector  OutputSignals=VRel,ARel
  Bus Selector1 : BusSelector  OutputSignals=VRel,ARel
  Bus Selector2 : BusSelector  OutputSignals=VRel,ARel
  CheckIfSolutionExists : Subsystem
  CheckIfSolutionExists1 : Subsystem
  Compare To Constant : simulink/Logic and Bit Operations/Compare To Constant  relop===  const=0  OutDataTypeStr=boolean
  Logical Operator : Logic  AllPortsSameDT=off  OutDataTypeStr=boolean
  Logop_1 : Logic  Operator=NOT  Inputs=1  AllPortsSameDT=off  OutDataTypeStr=boolean
  Logop_2 : Logic  Operator=NOT  Inputs=1  AllPortsSameDT=off  OutDataTypeStr=boolean
  Logop_3 : Logic  Inputs=3  AllPortsSameDT=off  OutDataTypeStr=boolean
  Switch_1 : Switch  Criteria=u2 ~= 0
  Switch_2 : Switch  Criteria=u2 ~= 0
  Switch_3 : Switch  Criteria=u2 ~= 0
  TiUpprLimIn : Constant  Value=P_TA_TiUpprLimInAsyEvlrCritEve
  TimeToIntersectionComputer : Subsystem
  TimeToIntersectionComputer1 : Subsystem  Commented=on
  MovingTTI : Outport
  MovingSolutionExists : Outport  Port=2
  wires:
  [MotionSetup1] -> Switch_1/1
  MotionDataNormalSetup/1 -> [MotionSetup1], Bus Selector1/1
  MotionDataAlternativeSetup/1 -> Switch_1/3, Bus Selector2/1
  Bus Selector2/2 -> CheckIfSolutionExists1/3(ARel)  "<ARel>"
  Bus Selector2/1 -> CheckIfSolutionExists1/2(VRel)  "<VRel>"
  Bus Selector1/2 -> CheckIfSolutionExists/3(ARel)  "<ARel>"
  Bus Selector1/1 -> CheckIfSolutionExists/2(VRel)  "<VRel>"
  CheckIfSolutionExists1/1(NoSolution) -> Logop_3/3  "NoSolutionMotionDataAlternativeSetup"
  CheckIfSolutionExists/1(NoSolution) -> Logop_3/1, Logical Operator/1  "NoSolutionMotionDataNormalSetup"
  TimeToIntersectionComputer/1(TimeToIntersection) -> Switch_3/3
  Bus Selector/2 -> TimeToIntersectionComputer/2(ARel)  "<ARel>"
  Bus Selector/1 -> TimeToIntersectionComputer/1(VRel)  "<VRel>"
  TiUpprLimIn/1 -> Switch_3/1  "DefaultTTI"
  Switch_3/1 -> MovingTTI/1  "MovingTTI"
  Switch_1/1 -> Bus Selector/1  "MotionData"
  EdgeDistanceNormalSetup/1 -> CheckIfSolutionExists/1(EdgeDistance), [EdgeSetup1]
  EdgeDistanceAlternativeSetup/1 -> CheckIfSolutionExists1/1(EdgeDistance), [EdgeSetup2]
  Logop_1/1 -> [SolutionSetup1]  "SolutionExistsForNormalSetup"
  [SolutionSetup1] -> Switch_1/2
  [SolutionSetup1] -> Switch_2/2
  Switch_2/1 -> TimeToIntersectionComputer/3(Posn)  "EdgeDistance"
  [EdgeSetup1] -> Switch_2/1
  [EdgeSetup2] -> Switch_2/3
  Logop_3/1 -> Logop_2/1, Switch_3/2  "NoSolution"
  Logop_2/1 -> MovingSolutionExists/1  "MovingSolutionExists"
  AEB_State/1 -> [A], Compare To Constant/1
  Compare To Constant/1 -> Logop_3/2, Logical Operator/2
  [A] -> TimeToIntersectionComputer/4(AEB_State)
  Logical Operator/1 -> Logop_1/1

== system TosAeb/AEB_Objsel/TA_Processor/AEB_ObjSelect/TargetInfoCheck/Data_precess/TA_EffectCalculate/Obj_FilterFlgCalculate/Long_ObjFilter/TTR_Calculate/CalculateTTRAndTTM_else/LongitudinalTTRComputer/CalculateTTI/StationaryHost ==
  EdgeDistanceNormalSetup : Inport
  MotionDataNormalSetup : Inport  Port=2
  EdgeDistanceAlternativeSetup : Inport  Port=3
  MotionDataAlternativeSetup : Inport  Port=4
  AEB_State : Inport  Port=5
  StoppingData : Inport  Port=6
  Bus Selector : BusSelector  OutputSignals=HostDeltaPos
  Bus Selector2 : BusSelector  OutputSignals=VRelStationaryHost,ARelStationaryHost
  Bus Selector3 : BusSelector  OutputSignals=VRelStationaryHost,ARelStationaryHost
  Bus Selector4 : BusSelector  OutputSignals=VRelStationaryHost,ARelStationaryHost
  CheckIfSolutionExists : Subsystem
  CheckIfSolutionExists1 : Subsystem
  Compare To Constant : simulink/Logic and Bit Operations/Compare To Constant  relop===  const=0  OutDataTypeStr=boolean
  Logical Operator : Logic  AllPortsSameDT=off  OutDataTypeStr=boolean
  Logop_1 : Logic  Operator=NOT  Inputs=1  AllPortsSameDT=off  OutDataTypeStr=boolean
  Logop_2 : Logic  Operator=NOT  Inputs=1  AllPortsSameDT=off  OutDataTypeStr=boolean
  Logop_4 : Logic  Inputs=3  AllPortsSameDT=off  OutDataTypeStr=boolean
  Subtract : Sum  Inputs=+-
  Switch_1 : Switch  Criteria=u2 ~= 0
  Switch_4 : Switch  Criteria=u2 ~= 0
  Switch_5 : Switch  Criteria=u2 ~= 0
  TiUpprLimIn1 : Constant  Value=P_TA_TiUpprLimInAsyEvlrCritEve
  TimeToIntersectionComputer : Subsystem
  TimeToIntersectionComputer1 : Subsystem  Commented=on
  StationaryHostTTI : Outport
  StationaryHostSolutionExists : Outport  Port=2
  wires:
  Bus Selector/1 -> Subtract/2  "<HostDeltaPos>"
  Subtract/1 -> TimeToIntersectionComputer/3(Posn)  "EdgeDistanceStationaryHost"
  Bus Selector3/2 -> CheckIfSolutionExists1/3(ARel)  "<ARelStationaryHost>"
  Bus Selector3/1 -> CheckIfSolutionExists1/2(VRel)  "<VRelStationaryHost>"
  Bus Selector2/2 -> CheckIfSolutionExists/3(ARel)  "<ARelStationaryHost>"
  Bus Selector2/1 -> CheckIfSolutionExists/2(VRel)  "<VRelStationaryHost>"
  EdgeDistanceAlternativeSetup/1 -> CheckIfSolutionExists1/1(EdgeDistance), [EdgeSetup2]
  EdgeDistanceNormalSetup/1 -> CheckIfSolutionExists/1(EdgeDistance), [EdgeSetup1]
  [EdgeSetup2] -> Switch_4/3
  [EdgeSetup1] -> Switch_4/1
  Switch_4/1 -> Subtract/1  "EdgeDistance"
  [SolutionSetup1] -> Switch_4/2
  [SolutionSetup1] -> Switch_1/2
  Switch_1/1 -> Bus Selector4/1  "MotionData"
  Switch_5/1 -> StationaryHostTTI/1  "StationaryHostTTI"
  TiUpprLimIn1/1 -> Switch_5/1  "DefaultTTI"
  Bus Selector4/1 -> TimeToIntersectionComputer/1(VRel)  "<VRelStationaryHost>"
  Bus Selector4/2 -> TimeToIntersectionComputer/2(ARel)  "<ARelStationaryHost>"
  TimeToIntersectionComputer/1(TimeToIntersection) -> Switch_5/3
  CheckIfSolutionExists/1(NoSolution) -> Logop_4/1, Logical Operator/1  "NoSolutionMotionDataNormalSetup"
  CheckIfSolutionExists1/1(NoSolution) -> Logop_4/3  "NoSolutionMotionDataAlternativeSetup"
  MotionDataAlternativeSetup/1 -> Bus Selector3/1, Switch_1/3
  [MotionSetup1] -> Switch_1/1
  StoppingData/1 -> Bus Selector/1
  MotionDataNormalSetup/1 -> Bus Selector2/1, [MotionSetup1]
  Logop_1/1 -> [SolutionSetup1]  "SolutionExistsForNormalSetup"
  Logop_4/1 -> Logop_2/1, Switch_5/2  "NoSolution"
  Logop_2/1 -> StationaryHostSolutionExists/1  "StationaryHostSolutionExists"
  AEB_State/1 -> [A], Compare To Constant/1
  Compare To Constant/1 -> Logical Operator/2, Logop_4/2
  [A] -> TimeToIntersectionComputer/4(AEB_State)
  Logical Operator/1 -> Logop_1/1

== system TosAeb/AEB_Objsel/TA_Processor/AEB_ObjSelect/TargetInfoCheck/Data_precess/TA_EffectCalculate/Obj_FilterFlgCalculate/Long_ObjFilter/TTR_Calculate/CalculateTTRAndTTM_else/LongitudinalTTRComputer/CalculateTTI/StationaryObject ==
  StoppingData : Inport
  EdgeDistanceNormalSetup : Inport  Port=2
  MotionDataNormalSetup : Inport  Port=3
  EdgeDistanceAlternativeSetup : Inport  Port=4
  MotionDataAlternativeSetup : Inport  Port=5
  AEB_State : Inport  Port=6
  Bus Selector : BusSelector  OutputSignals=ObjectDeltaPos
  Bus Selector1 : BusSelector  OutputSignals=VRelStationaryObject,ARelStationaryObject
  Bus Selector2 : BusSelector  OutputSignals=VRelStationaryObject,ARelStationaryObject
  Bus Selector3 : BusSelector  OutputSignals=VRelStationaryObject,ARelStationaryObject
  CheckIfSolutionExists : Subsystem
  CheckIfSolutionExists1 : Subsystem
  Compare To Constant : simulink/Logic and Bit Operations/Compare To Constant  relop===  const=0  OutDataTypeStr=boolean
  Logical Operator : Logic  AllPortsSameDT=off  OutDataTypeStr=boolean
  Logop_1 : Logic  Operator=NOT  Inputs=1  AllPortsSameDT=off  OutDataTypeStr=boolean
  Logop_2 : Logic  Operator=NOT  Inputs=1  AllPortsSameDT=off  OutDataTypeStr=boolean
  Logop_3 : Logic  Inputs=3  AllPortsSameDT=off  OutDataTypeStr=boolean
  Subtract : Sum
  Switch_1 : Switch  Criteria=u2 ~= 0
  Switch_3 : Switch  Criteria=u2 ~= 0
  Switch_4 : Switch  Criteria=u2 ~= 0
  TiUpprLimIn : Constant  Value=P_TA_TiUpprLimInAsyEvlrCritEve
  TimeToIntersectionComputer : Subsystem
  TimeToIntersectionComputer1 : Subsystem  Commented=on
  StationaryObjectTTI : Outport
  StationaryObjectSolutionExists : Outport  Port=2
  wires:
  Switch_4/1 -> Subtract/1  "EdgeDistance"
  EdgeDistanceAlternativeSetup/1 -> CheckIfSolutionExists1/1(EdgeDistance), [EdgeSetup2]
  EdgeDistanceNormalSetup/1 -> CheckIfSolutionExists/1(EdgeDistance), [EdgeSetup1]
  [EdgeSetup2] -> Switch_4/3
  [EdgeSetup1] -> Switch_4/1
  [SolutionSetup1] -> Switch_4/2
  [SolutionSetup1] -> Switch_1/2
  [MotionSetup1] -> Switch_1/1
  Switch_3/1 -> StationaryObjectTTI/1  "StationaryObjectTTI"
  Subtract/1 -> TimeToIntersectionComputer/3(Posn)  "EdgeDistanceStationaryObject"
  Bus Selector/1 -> Subtract/2  "<ObjectDeltaPos>"
  StoppingData/1 -> Bus Selector/1
  Switch_1/1 -> Bus Selector1/1  "MotionData"
  Logop_3/1 -> Logop_2/1, Switch_3/2  "NoSolution"
  TiUpprLimIn/1 -> Switch_3/1  "DefaultTTI"
  Bus Selector1/1 -> TimeToIntersectionComputer/1(VRel)  "<VRelStationaryObject>"
  Bus Selector1/2 -> TimeToIntersectionComputer/2(ARel)  "<ARelStationaryObject>"
  TimeToIntersectionComputer/1(TimeToIntersection) -> Switch_3/3
  CheckIfSolutionExists/1(NoSolution) -> Logop_3/1, Logical Operator/1  "NoSolutionMotionDataNormalSetup"
  CheckIfSolutionExists1/1(NoSolution) -> Logop_3/3  "NoSolutionMotionDataAlternativeSetup"
  Bus Selector2/1 -> CheckIfSolutionExists/2(VRel)  "<VRelStationaryObject>"
  Bus Selector2/2 -> CheckIfSolutionExists/3(ARel)  "<ARelStationaryObject>"
  Bus Selector3/1 -> CheckIfSolutionExists1/2(VRel)  "<VRelStationaryObject>"
  Bus Selector3/2 -> CheckIfSolutionExists1/3(ARel)  "<ARelStationaryObject>"
  MotionDataAlternativeSetup/1 -> Bus Selector3/1, Switch_1/3
  MotionDataNormalSetup/1 -> Bus Selector2/1, [MotionSetup1]
  Logop_1/1 -> [SolutionSetup1]  "SolutionExistsForNormalSetup"
  Logop_2/1 -> StationaryObjectSolutionExists/1  "StationaryObjectSolutionExists"
  AEB_State/1 -> Compare To Constant/1, [A]
  Compare To Constant/1 -> Logop_3/2, Logical Operator/2
  [A] -> TimeToIntersectionComputer/4(AEB_State)
  Logical Operator/1 -> Logop_1/1

== system TosAeb/AEB_Objsel/TA_Processor/AEB_ObjSelect/TargetInfoCheck/Data_precess/TA_EffectCalculate/Obj_FilterFlgCalculate/Long_ObjFilter/TTR_Calculate/CalculateTTRAndTTM_else/LongitudinalTTRComputer/DetermineLongitudinalClosestSide/Compare To Zero1 ==
  u : Inport
  Compare : RelationalOperator  Operator=<  OutDataTypeStr=boolean  RndMeth=Nearest  InputSameDT=on
  Constant : Constant  DisableCoverage=on  Value=0  OutDataTypeStr=Inherit: Inherit via back propagation  PreserveConstantTs=on
  y : Outport
  wires:
  Constant/1 -> Compare/2
  u/1 -> Compare/1
  Compare/1 -> y/1

== system TosAeb/AEB_Objsel/TA_Processor/AEB_ObjSelect/TargetInfoCheck/Data_precess/TA_EffectCalculate/Obj_FilterFlgCalculate/Long_ObjFilter/TTR_Calculate/CalculateTTRAndTTM_else/LongitudinalTTRComputer/DetermineLongitudinalClosestSide/Compare To Zero5 ==
  u : Inport
  Compare : RelationalOperator  Operator=>  OutDataTypeStr=boolean  RndMeth=Nearest  InputSameDT=on
  Constant : Constant  DisableCoverage=on  Value=0  OutDataTypeStr=Inherit: Inherit via back propagation  PreserveConstantTs=on
  y : Outport
  wires:
  Constant/1 -> Compare/2
  u/1 -> Compare/1
  Compare/1 -> y/1

== system TosAeb/AEB_Objsel/TA_Processor/AEB_ObjSelect/TargetInfoCheck/Data_precess/TA_EffectCalculate/Obj_FilterFlgCalculate/Long_ObjFilter/TTR_Calculate/CalculateTTRAndTTM_else/LongitudinalTTRComputer/DetermineLongitudinalClosestSide/SizeChecker ==
  note: 0.01
  Signal : Inport  OutDataTypeStr=DataTypeIn
      settings: AttributesFormatString=DT=%<OutDataTypeStr>
  Abs : Abs  SaturateOnIntegerOverflow=on
  LimitSource : Constant  Value=Limit  OutDataTypeStr=Inherit: Inherit via back propagation
      settings: AttributesFormatString=DT=%<OutDataTypeStr>
  Relational Operator : RelationalOperator  Operator=>  OutDataTypeStr=boolean  RndMeth=Nearest  InputSameDT=on
      settings: AttributesFormatString=InputSameDT=%<InputSameDT>
  TestOk : Outport  OutDataTypeStr=boolean
      settings: AttributesFormatString=DT=%<OutDataTypeStr>
  wires:
  Relational Operator/1 -> TestOk/1  "TestOk"
  Signal/1 -> Abs/1  "Signal"
  LimitSource/1 -> Relational Operator/2  "LimitSource"
  Abs/1 -> Relational Operator/1  "AbsoluteSignal"

== system TosAeb/AEB_Objsel/TA_Processor/AEB_ObjSelect/TargetInfoCheck/Data_precess/TA_EffectCalculate/Obj_FilterFlgCalculate/Long_ObjFilter/TTR_Calculate/CalculateTTRAndTTM_else/LongitudinalTTRComputer/SelectTTI/BothHostAndObjectWillStop ==
  note: If both host and object will stop,
      | we use the TTI calculated with the shortest stop time.
  StationaryObjectTTI : Inport
  StationaryHostTTI : Inport  Port=2
  ObjectStopTime : Inport  Port=3
  HostStopTime : Inport  Port=4
  StationaryObjectSolutionExists : Inport  Port=5
  StationaryHostSolutionExists : Inport  Port=6
  Action Port : ActionPort  ActionPortLabel=if(u1~=0)
  Relop_3 : RelationalOperator  Operator=<  OutDataTypeStr=boolean  RndMeth=Nearest
  Switch_1 : Switch  Criteria=u2 ~= 0
  Switch_2 : Switch  Criteria=u2 ~= 0
  TimeToIntersection : Outport
  SolutionExists : Outport  Port=2
  wires:
  StationaryHostSolutionExists/1 -> Switch_2/3
  StationaryObjectSolutionExists/1 -> Switch_2/1
  StationaryHostTTI/1 -> Switch_1/3
  StationaryObjectTTI/1 -> Switch_1/1
  Relop_3/1 -> [ObjectStops], Switch_1/2  "ObjectStopTimeIsShortest"
  HostStopTime/1 -> Relop_3/2
  ObjectStopTime/1 -> Relop_3/1
  Switch_1/1 -> TimeToIntersection/1  "TimeToIntersection"
  Switch_2/1 -> SolutionExists/1  "SolutionExists"
  [ObjectStops] -> Switch_2/2

== system TosAeb/AEB_Objsel/TA_Processor/AEB_ObjSelect/TargetInfoCheck/Data_precess/TA_EffectCalculate/Obj_FilterFlgCalculate/Long_ObjFilter/TTR_Calculate/CalculateTTRAndTTM_else/LongitudinalTTRComputer/SelectTTI/CheckIfVehicleStops ==
  note: Maximum prediction time for object position (longitudinal threat assessor)
  note: 3
  note: The Stationary TTI is valid if the other vehicle reaches the position where this vehicle stops,
      | at a time that occurs after this vehicle has stopped.
  note: If MovingTTI is positive and smaller than StopTime, the intersection occurs before the vehicle stops.
  StationaryTTI : Inport
  MovingTTI : Inport  Port=2
  StopTime : Inport  Port=3
  Compare To Zero1 : Subsystem  mask(Compare To Zero){relop=>, OutDataTypeStr=boolean, ZeroCross=on}
      mask prompts: relop "Simulink:masks:Operator_MP"; OutDataTypeStr "Simulink:masks:OutputDataType_MP"; ZeroCross "Simulink:masks:EnableZerocrossingDetection_MP"
      mask help: Simulink:masks:CompareToZero_MD
      mask init: same as TosAeb/AEB_Objsel/TA_Processor/AEB_ObjSelect/TargetInfoCheck/Data_precess/TA_EffectCalculate/Obj_FilterFlgCalculate/Long_ObjFilter/TTR_Calculate/CalculateTTRAndTTM_else/ModifyTTRAtSameSpeedAndAcceleration/Compare To Zero
      mask TypeOptions relop: == | ~= | < | <= | >= | >
      mask TypeOptions OutDataTypeStr: Simulink:masks:uint8 | Simulink:masks:boolean
      mask Callback ZeroCross: sl('zeroCrossEnable', gcbh);
      mask Display: disp([relop,' 0'])
  Compare To Zero2 : Subsystem  mask(Compare To Zero){relop=>, OutDataTypeStr=boolean, ZeroCross=on}
      mask prompts: relop "Simulink:masks:Operator_MP"; OutDataTypeStr "Simulink:masks:OutputDataType_MP"; ZeroCross "Simulink:masks:EnableZerocrossingDetection_MP"
      mask help: Simulink:masks:CompareToZero_MD
      mask init: same as TosAeb/AEB_Objsel/TA_Processor/AEB_ObjSelect/TargetInfoCheck/Data_precess/TA_EffectCalculate/Obj_FilterFlgCalculate/Long_ObjFilter/TTR_Calculate/CalculateTTRAndTTM_else/ModifyTTRAtSameSpeedAndAcceleration/Compare To Zero
      mask TypeOptions relop: == | ~= | < | <= | >= | >
      mask TypeOptions OutDataTypeStr: Simulink:masks:uint8 | Simulink:masks:boolean
      mask Callback ZeroCross: sl('zeroCrossEnable', gcbh);
      mask Display: disp([relop,' 0'])
  Logop_1 : Logic  AllPortsSameDT=off  OutDataTypeStr=boolean
  Logop_2 : Logic  Inputs=4  AllPortsSameDT=off  OutDataTypeStr=boolean
  Logop_3 : Logic  Operator=NOT  Inputs=1  AllPortsSameDT=off  OutDataTypeStr=boolean
  Relop_1 : RelationalOperator  Operator=>  OutDataTypeStr=boolean  RndMeth=Nearest
  Relop_2 : RelationalOperator  Operator=>  OutDataTypeStr=boolean  RndMeth=Nearest
  Relop_4 : RelationalOperator  Operator=<  OutDataTypeStr=boolean  RndMeth=Nearest
  SftyArUpprLimForTiInObjPred : Constant  Value=P_TA_SftyArUpprLimForTi
  VehicleStops : Outport
  wires:
  Relop_1/1 -> Logop_2/1  "StationaryTTIValid"
  StopTime/1 -> Relop_1/2, Compare To Zero1/1(u), Relop_4/1, Relop_2/1  "StopTime"
  StationaryTTI/1 -> Relop_1/1  "StationaryTTI"
  Logop_2/1 -> VehicleStops/1  "VehicleStops"
  Relop_4/1 -> Logop_2/3  "StopsWithinPredictionTime"
  Compare To Zero1/1(y) -> Logop_2/2  "StopsAtAll"
  SftyArUpprLimForTiInObjPred/1 -> Relop_4/2
  Relop_2/1 -> Logop_1/1
  MovingTTI/1 -> Compare To Zero2/1(u), Relop_2/2  "MovingTTI"
  Compare To Zero2/1(y) -> Logop_1/2
  Logop_1/1 -> Logop_3/1  "IntersectionBeforeVehicleStops"
  Logop_3/1 -> Logop_2/4  "StopsBeforeIntersection"

== system TosAeb/AEB_Objsel/TA_Processor/AEB_ObjSelect/TargetInfoCheck/Data_precess/TA_EffectCalculate/Obj_FilterFlgCalculate/Long_ObjFilter/TTR_Calculate/CalculateTTRAndTTM_else/LongitudinalTTRComputer/SelectTTI/CheckIfVehicleStops1 ==
  note: 3
  note: The Stationary TTI is valid if the other vehicle reaches the position where this vehicle stops,
      | at a time that occurs after this vehicle has stopped.
  note: If MovingTTI is positive and smaller than StopTime, the intersection occurs before the vehicle stops.
  StationaryTTI : Inport
  MovingTTI : Inport  Port=2
  StopTime : Inport  Port=3
  Compare To Zero1 : Subsystem  mask(Compare To Zero){relop=>, OutDataTypeStr=boolean, ZeroCross=on}
      mask prompts: relop "Simulink:masks:Operator_MP"; OutDataTypeStr "Simulink:masks:OutputDataType_MP"; ZeroCross "Simulink:masks:EnableZerocrossingDetection_MP"
      mask help: Simulink:masks:CompareToZero_MD
      mask init: same as TosAeb/AEB_Objsel/TA_Processor/AEB_ObjSelect/TargetInfoCheck/Data_precess/TA_EffectCalculate/Obj_FilterFlgCalculate/Long_ObjFilter/TTR_Calculate/CalculateTTRAndTTM_else/ModifyTTRAtSameSpeedAndAcceleration/Compare To Zero
      mask TypeOptions relop: == | ~= | < | <= | >= | >
      mask TypeOptions OutDataTypeStr: Simulink:masks:uint8 | Simulink:masks:boolean
      mask Callback ZeroCross: sl('zeroCrossEnable', gcbh);
      mask Display: disp([relop,' 0'])
  Compare To Zero2 : Subsystem  mask(Compare To Zero){relop=>, OutDataTypeStr=boolean, ZeroCross=on}
      mask prompts: relop "Simulink:masks:Operator_MP"; OutDataTypeStr "Simulink:masks:OutputDataType_MP"; ZeroCross "Simulink:masks:EnableZerocrossingDetection_MP"
      mask help: Simulink:masks:CompareToZero_MD
      mask init: same as TosAeb/AEB_Objsel/TA_Processor/AEB_ObjSelect/TargetInfoCheck/Data_precess/TA_EffectCalculate/Obj_FilterFlgCalculate/Long_ObjFilter/TTR_Calculate/CalculateTTRAndTTM_else/ModifyTTRAtSameSpeedAndAcceleration/Compare To Zero
      mask TypeOptions relop: == | ~= | < | <= | >= | >
      mask TypeOptions OutDataTypeStr: Simulink:masks:uint8 | Simulink:masks:boolean
      mask Callback ZeroCross: sl('zeroCrossEnable', gcbh);
      mask Display: disp([relop,' 0'])
  Logop_1 : Logic  AllPortsSameDT=off  OutDataTypeStr=boolean
  Logop_2 : Logic  Inputs=4  AllPortsSameDT=off  OutDataTypeStr=boolean
  Logop_3 : Logic  Operator=NOT  Inputs=1  AllPortsSameDT=off  OutDataTypeStr=boolean
  Relop_1 : RelationalOperator  Operator=>  OutDataTypeStr=boolean  RndMeth=Nearest
  Relop_2 : RelationalOperator  Operator=>  OutDataTypeStr=boolean  RndMeth=Nearest
  Relop_4 : RelationalOperator  Operator=<  OutDataTypeStr=boolean  RndMeth=Nearest
  SftyArUpprLimForTiInObjPred : Constant  Value=P_TA_SftyArUpprLimForTi
  VehicleStops : Outport
  wires:
  Relop_1/1 -> Logop_2/1  "StationaryTTIValid"
  StopTime/1 -> Relop_1/2, Compare To Zero1/1(u), Relop_4/1, Relop_2/1  "StopTime"
  StationaryTTI/1 -> Relop_1/1  "StationaryTTI"
  Logop_2/1 -> VehicleStops/1  "VehicleStops"
  Relop_4/1 -> Logop_2/3  "StopsWithinPredictionTime"
  Compare To Zero1/1(y) -> Logop_2/2  "StopsAtAll"
  SftyArUpprLimForTiInObjPred/1 -> Relop_4/2
  Relop_2/1 -> Logop_1/1
  MovingTTI/1 -> Compare To Zero2/1(u), Relop_2/2  "MovingTTI"
  Compare To Zero2/1(y) -> Logop_1/2
  Logop_1/1 -> Logop_3/1  "IntersectionBeforeVehicleStops"
  Logop_3/1 -> Logop_2/4  "StopsBeforeIntersection"

== system TosAeb/AEB_Objsel/TA_Processor/AEB_ObjSelect/TargetInfoCheck/Data_precess/TA_EffectCalculate/Obj_FilterFlgCalculate/Long_ObjFilter/TTR_Calculate/CalculateTTRAndTTM_else/LongitudinalTTRComputer/SelectTTI/HostAndObjectAreMoving ==
  MovingTTI : Inport
  MovingSolutionExists : Inport  Port=2
  Action Port : ActionPort  ActionPortLabel=else
  NoOperation : Subsystem  mask(noop){}
      mask help: No operation block to to hadle when a signal is passed unchanged between ports with different names
      mask Display: disp('NOOP')
  NoOperation1 : Subsystem  mask(noop){}
      mask help: No operation block to to hadle when a signal is passed unchanged between ports with different names
      mask Display: disp('NOOP')
  TimeToIntersection : Outport
  SolutionExists : Outport  Port=2
  wires:
  NoOperation/1(Out) -> TimeToIntersection/1  "TimeToIntersection"
  MovingTTI/1 -> NoOperation/1(In)
  NoOperation1/1(Out) -> SolutionExists/1  "SolutionExists"
  MovingSolutionExists/1 -> NoOperation1/1(In)

== system TosAeb/AEB_Objsel/TA_Processor/AEB_ObjSelect/TargetInfoCheck/Data_precess/TA_EffectCalculate/Obj_FilterFlgCalculate/Long_ObjFilter/TTR_Calculate/CalculateTTRAndTTM_else/LongitudinalTTRComputer/SelectTTI/HostOnlyWillStop ==
  StationaryHostTTI : Inport
  StationaryHostSolutionExists : Inport  Port=2
  Action Port : ActionPort  ActionPortLabel=elseif(u2~=0)
  NoOperation : Subsystem  mask(noop){}
      mask help: No operation block to to hadle when a signal is passed unchanged between ports with different names
      mask Display: disp('NOOP')
  NoOperation1 : Subsystem  mask(noop){}
      mask help: No operation block to to hadle when a signal is passed unchanged between ports with different names
      mask Display: disp('NOOP')
  TimeToIntersection : Outport
  SolutionExists : Outport  Port=2
  wires:
  StationaryHostTTI/1 -> NoOperation/1(In)
  NoOperation/1(Out) -> TimeToIntersection/1  "TimeToIntersection"
  NoOperation1/1(Out) -> SolutionExists/1  "SolutionExists"
  StationaryHostSolutionExists/1 -> NoOperation1/1(In)
